simulink model "gate_V37_2016a_HIL_C"  (R2016a)
settings: Solver=FixedStepDiscrete  StartTime=0.0  StopTime=120  FixedStep=0.001  RelTol=1e-3
values from neighbouring files (symbols this model uses):
  CLOSE_SIDE_VALUE = single(4*ONE_SEC)    [GateParameter.m]
  CLOSED = uint8(12)    [GateParameter.m]
  CLOSING = uint8(13)    [GateParameter.m]
  CURRENT_STABLE_TIMER = uint8(200)    [GateParameter.m]
  CURRENT_THRESH = uint16(1000)    [GateParameter.m]
  INIT = uint8(15)    [GateParameter.m]
  MOVING_TIMER = uint32(60*ONE_SEC)    [GateParameter.m]
  OPEN = uint8(14)    [GateParameter.m]
  OPEN_SIDE_VALUE = uint16(4*ONE_SEC)    [GateParameter.m]
  OPENING = uint8(11)    [GateParameter.m]
  OPENING_ACTIVE = uint8(1)    [GateParameter.m]
  STABLE_TIMER_VALUE = uint16(1*ONE_SEC)    [GateParameter.m]
undefined symbols (not defined in the file or in its folder): sigbuilder_block
libraries: simulink x9, arduinolib x7
(part 2 of 2)

== system gate_V37_2016a_HIL_C/Gate/Subsystem/C_HardwareOutputWrapper_RF/MotorHandler ==
  leftMotor : Inport
  rightMotor : Inport  Port=2
  gateState : Inport  Port=3
  CLOSED : Constant  Value=CLOSED  SampleTime=-1
  CLOSING : Constant  Value=CLOSING  SampleTime=-1
  MotorsHandler : Stateflow chart  TreatAsAtomicUnit=on
  OPEN : Constant  Value=OPEN  SampleTime=-1
  OPENING : Constant  Value=OPENING  SampleTime=-1
  OPENING1 : Constant  Value=INIT  SampleTime=-1
  LeftPin : Outport
  ComPin : Outport  Port=2
  RightPin : Outport  Port=3
  wires:
  OPENING1/1 -> MotorsHandler/8(INIT)
  leftMotor/1 -> MotorsHandler/1(leftMotor)
  gateState/1 -> MotorsHandler/7(gateState)
  MotorsHandler/3(RightMotorPin_BOOL) -> RightPin/1
  MotorsHandler/2(ComMotorPin_BOOL) -> ComPin/1
  MotorsHandler/1(LeftMotorPin_BOOL) -> LeftPin/1
  OPENING/1 -> MotorsHandler/6(OPENING)
  OPEN/1 -> MotorsHandler/5(OPEN)
  CLOSING/1 -> MotorsHandler/4(CLOSING)
  CLOSED/1 -> MotorsHandler/3(CLOSED)
  rightMotor/1 -> MotorsHandler/2(rightMotor)

== system gate_V37_2016a_HIL_C/Gate/Subsystem/CurrentFilter/Subsystem ==
  rightCurrentRaw : Inport
  leftCurrentRaw : Inport  Port=2
  Data Type Conversion : DataTypeConversion  OutDataTypeStr=uint16
  Data Type Conversion1 : DataTypeConversion  OutDataTypeStr=uint16
  Logical Operator : Logic  Operator=OR  AllPortsSameDT=off  OutDataTypeStr=boolean
  S-Function Builder2 : S-Function  Commented=on  InitFcn=try, set_param(gcb,'FunctionName','printingBlockMotor'), end  FunctionName=printingBlockMotor  SFunctionModules=printingBlockMotor_wrapper  SFunctionDeploymentMode=off  EnableBusSupport=off  mask(S-Function Builder){}
      mask help: Simulink:masks:SFunctionBuilder_MD
      mask init: same as gate_V37_2016a_HIL_C/Gate/HardwareInputWrapper/HIL_B/S-Function Builder1
      settings: CopyFcn=set_param(gcbh,'LinkStatus','none')  PreSaveFcn=try, set_param(gcb,'FunctionName','printingBlockMotor'), end  OpenFcn=sfunctionwizard(gcbh)
      mask Display: plot(val(:,1),val(:,2)),disp(sys), port_label('input',1,'u0'), port_label('input',2,'u1')
  Unit Delay : UnitDelay  SampleTime=-1
  Unit Delay1 : UnitDelay  SampleTime=-1
  WRONG_PASSWORD : Constant  Value=CURRENT_STABLE_TIMER  SampleTime=-1
  WRONG_PASSWORD1 : Constant  Value=CURRENT_STABLE_TIMER  SampleTime=-1
  WRONG_PASSWORD2 : Constant  Value=CURRENT_THRESH  SampleTime=-1
  WRONG_PASSWORD3 : Constant  Value=CURRENT_THRESH  SampleTime=-1
  leftSquareWaveDetector : Stateflow chart  TreatAsAtomicUnit=on
  rightSquareWaveDetector : Stateflow chart  TreatAsAtomicUnit=on
  current : Outport
  wires:
  WRONG_PASSWORD3/1 -> leftSquareWaveDetector/4(CURRENT_THRESH)
  WRONG_PASSWORD2/1 -> rightSquareWaveDetector/4(CURRENT_THRESH)
  WRONG_PASSWORD1/1 -> leftSquareWaveDetector/3(CURRENT_STABLE_TIMER)
  WRONG_PASSWORD/1 -> rightSquareWaveDetector/3(CURRENT_STABLE_TIMER)
  Data Type Conversion1/1 -> S-Function Builder2/2
  Data Type Conversion/1 -> S-Function Builder2/1
  Logical Operator/1 -> current/1
  leftSquareWaveDetector/1(currentValue) -> Data Type Conversion1/1, Logical Operator/2
  rightSquareWaveDetector/1(currentValue) -> Logical Operator/1, Data Type Conversion/1
  Unit Delay1/1 -> leftSquareWaveDetector/2(CurrentAnalogInOld)
  leftCurrentRaw/1 -> leftSquareWaveDetector/1(CurrentAnalogIn), Unit Delay1/1
  Unit Delay/1 -> rightSquareWaveDetector/2(CurrentAnalogInOld)
  rightCurrentRaw/1 -> Unit Delay/1, rightSquareWaveDetector/1(CurrentAnalogIn)

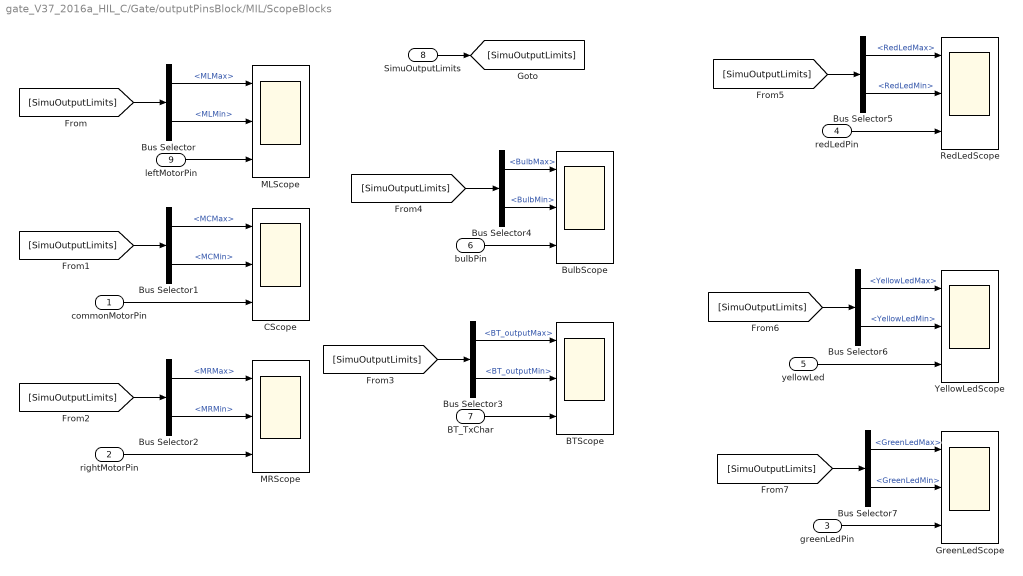
== system gate_V37_2016a_HIL_C/Gate/outputPinsBlock/MIL/ScopeBlocks ==
  commonMotorPin : Inport
  rightMotorPin : Inport  Port=2
  greenLedPin : Inport  Port=3
  redLedPin : Inport  Port=4
  yellowLed : Inport  Port=5
  bulbPin : Inport  Port=6
  BT_TxChar : Inport  Port=7
  SimuOutputLimits : Inport  Port=8
  leftMotorPin : Inport  Port=9
  BTScope : Scope  NumInputPorts=3
  BulbScope : Scope  NumInputPorts=3
  Bus Selector : BusSelector  OutputSignals=MLMax,MLMin
  Bus Selector1 : BusSelector  OutputSignals=MCMax,MCMin
  Bus Selector2 : BusSelector  OutputSignals=MRMax,MRMin
  Bus Selector3 : BusSelector  OutputSignals=BT_outputMax,BT_outputMin
  Bus Selector4 : BusSelector  OutputSignals=BulbMax,BulbMin
  Bus Selector5 : BusSelector  OutputSignals=RedLedMax,RedLedMin
  Bus Selector6 : BusSelector  OutputSignals=YellowLedMax,YellowLedMin
  Bus Selector7 : BusSelector  OutputSignals=GreenLedMax,GreenLedMin
  CScope : Scope  NumInputPorts=3
  GreenLedScope : Scope  NumInputPorts=3
  MLScope : Scope  NumInputPorts=3
  MRScope : Scope  NumInputPorts=3
  RedLedScope : Scope  NumInputPorts=3
  YellowLedScope : Scope  NumInputPorts=3
  wires:
  rightMotorPin/1 -> MRScope/3
  commonMotorPin/1 -> CScope/3
  bulbPin/1 -> BulbScope/3
  greenLedPin/1 -> GreenLedScope/3
  yellowLed/1 -> YellowLedScope/3
  redLedPin/1 -> RedLedScope/3
  BT_TxChar/1 -> BTScope/3
  SimuOutputLimits/1 -> [SimuOutputLimits]
  [SimuOutputLimits] -> Bus Selector/1
  leftMotorPin/1 -> MLScope/3
  Bus Selector/1 -> MLScope/1  "<MLMax>"
  Bus Selector/2 -> MLScope/2  "<MLMin>"
  [SimuOutputLimits] -> Bus Selector1/1
  Bus Selector1/1 -> CScope/1  "<MCMax>"
  Bus Selector1/2 -> CScope/2  "<MCMin>"
  [SimuOutputLimits] -> Bus Selector2/1
  Bus Selector2/1 -> MRScope/1  "<MRMax>"
  Bus Selector2/2 -> MRScope/2  "<MRMin>"
  [SimuOutputLimits] -> Bus Selector3/1
  Bus Selector3/1 -> BTScope/1  "<BT_outputMax>"
  Bus Selector3/2 -> BTScope/2  "<BT_outputMin>"
  [SimuOutputLimits] -> Bus Selector4/1
  Bus Selector4/1 -> BulbScope/1  "<BulbMax>"
  Bus Selector4/2 -> BulbScope/2  "<BulbMin>"
  [SimuOutputLimits] -> Bus Selector5/1
  Bus Selector5/1 -> RedLedScope/1  "<RedLedMax>"
  Bus Selector5/2 -> RedLedScope/2  "<RedLedMin>"
  [SimuOutputLimits] -> Bus Selector6/1
  Bus Selector6/1 -> YellowLedScope/1  "<YellowLedMax>"
  Bus Selector6/2 -> YellowLedScope/2  "<YellowLedMin>"
  [SimuOutputLimits] -> Bus Selector7/1
  Bus Selector7/1 -> GreenLedScope/1  "<GreenLedMax>"
  Bus Selector7/2 -> GreenLedScope/2  "<GreenLedMin>"

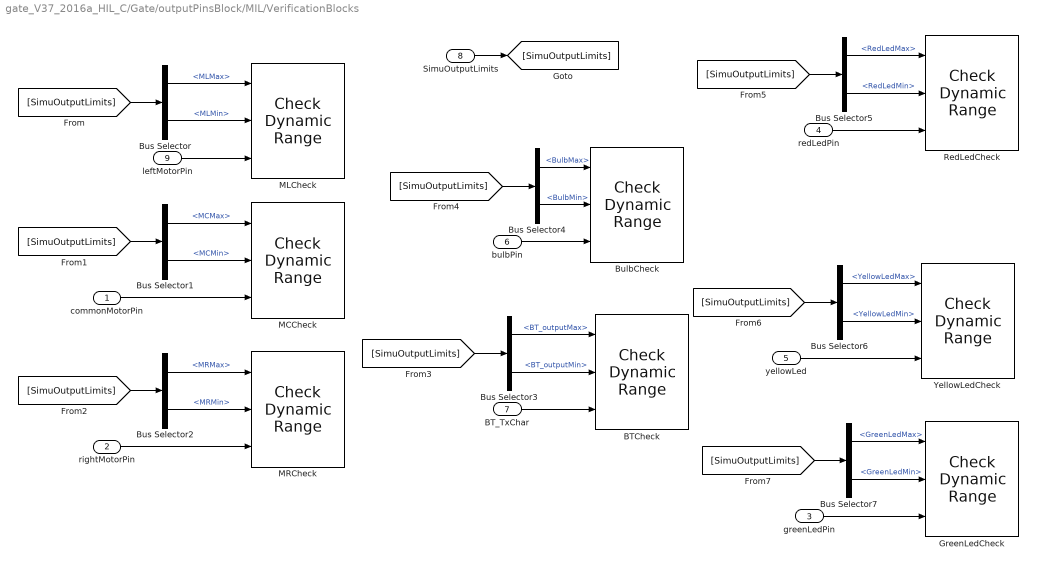
== system gate_V37_2016a_HIL_C/Gate/outputPinsBlock/MIL/VerificationBlocks  ==
  commonMotorPin : Inport
  rightMotorPin : Inport  Port=2
  greenLedPin : Inport  Port=3
  redLedPin : Inport  Port=4
  yellowLed : Inport  Port=5
  bulbPin : Inport  Port=6
  BT_TxChar : Inport  Port=7
  SimuOutputLimits : Inport  Port=8
  leftMotorPin : Inport  Port=9
  BTCheck : simulink/Model Verification/Check  Dynamic Range  Description=min < sig < max  enabled=on  stopWhenAssertionFail=on  export=off  icon=graphic
  BulbCheck : simulink/Model Verification/Check  Dynamic Range  Description=min < sig < max  enabled=on  stopWhenAssertionFail=on  export=off  icon=graphic
  Bus Selector : BusSelector  OutputSignals=MLMax,MLMin
  Bus Selector1 : BusSelector  OutputSignals=MCMax,MCMin
  Bus Selector2 : BusSelector  OutputSignals=MRMax,MRMin
  Bus Selector3 : BusSelector  OutputSignals=BT_outputMax,BT_outputMin
  Bus Selector4 : BusSelector  OutputSignals=BulbMax,BulbMin
  Bus Selector5 : BusSelector  OutputSignals=RedLedMax,RedLedMin
  Bus Selector6 : BusSelector  OutputSignals=YellowLedMax,YellowLedMin
  Bus Selector7 : BusSelector  OutputSignals=GreenLedMax,GreenLedMin
  GreenLedCheck : simulink/Model Verification/Check  Dynamic Range  Description=min < sig < max  enabled=on  stopWhenAssertionFail=on  export=off  icon=graphic
  MCCheck : simulink/Model Verification/Check  Dynamic Range  Description=min < sig < max  enabled=on  stopWhenAssertionFail=on  export=off  icon=graphic
  MLCheck : simulink/Model Verification/Check  Dynamic Range  Description=min < sig < max  enabled=on  stopWhenAssertionFail=on  export=off  icon=graphic
  MRCheck : simulink/Model Verification/Check  Dynamic Range  Description=min < sig < max  enabled=on  stopWhenAssertionFail=on  export=off  icon=graphic
  RedLedCheck : simulink/Model Verification/Check  Dynamic Range  Description=min < sig < max  enabled=on  stopWhenAssertionFail=on  export=off  icon=graphic
  YellowLedCheck : simulink/Model Verification/Check  Dynamic Range  Description=min < sig < max  enabled=on  stopWhenAssertionFail=on  export=off  icon=graphic
  wires:
  rightMotorPin/1 -> MRCheck/3
  commonMotorPin/1 -> MCCheck/3
  bulbPin/1 -> BulbCheck/3
  greenLedPin/1 -> GreenLedCheck/3
  yellowLed/1 -> YellowLedCheck/3
  redLedPin/1 -> RedLedCheck/3
  BT_TxChar/1 -> BTCheck/3
  SimuOutputLimits/1 -> [SimuOutputLimits]
  [SimuOutputLimits] -> Bus Selector/1
  leftMotorPin/1 -> MLCheck/3
  Bus Selector/1 -> MLCheck/1  "<MLMax>"
  Bus Selector/2 -> MLCheck/2  "<MLMin>"
  [SimuOutputLimits] -> Bus Selector1/1
  Bus Selector1/1 -> MCCheck/1  "<MCMax>"
  Bus Selector1/2 -> MCCheck/2  "<MCMin>"
  [SimuOutputLimits] -> Bus Selector2/1
  Bus Selector2/1 -> MRCheck/1  "<MRMax>"
  Bus Selector2/2 -> MRCheck/2  "<MRMin>"
  [SimuOutputLimits] -> Bus Selector3/1
  Bus Selector3/1 -> BTCheck/1  "<BT_outputMax>"
  Bus Selector3/2 -> BTCheck/2  "<BT_outputMin>"
  [SimuOutputLimits] -> Bus Selector4/1
  Bus Selector4/1 -> BulbCheck/1  "<BulbMax>"
  Bus Selector4/2 -> BulbCheck/2  "<BulbMin>"
  [SimuOutputLimits] -> Bus Selector5/1
  Bus Selector5/1 -> RedLedCheck/1  "<RedLedMax>"
  Bus Selector5/2 -> RedLedCheck/2  "<RedLedMin>"
  [SimuOutputLimits] -> Bus Selector6/1
  Bus Selector6/1 -> YellowLedCheck/1  "<YellowLedMax>"
  Bus Selector6/2 -> YellowLedCheck/2  "<YellowLedMin>"
  [SimuOutputLimits] -> Bus Selector7/1
  Bus Selector7/1 -> GreenLedCheck/1  "<GreenLedMax>"
  Bus Selector7/2 -> GreenLedCheck/2  "<GreenLedMin>"

== system gate_V37_2016a_HIL_C/Gate/HardwareInputWrapper/HIL_B/EEPROM/EEPROM_UPDATE ==
  clearReq : Inport
  readReq : Inport  Port=2
  writeReq : Inport  Port=3
  passwordIn : Inport  Port=4
  passAssignedIn : Inport  Port=5
  S-Function Builder : S-Function  InitFcn=try, set_param(gcb,'FunctionName','myEEPROM_Handler'), end  FunctionName=myEEPROM_Handler  SFunctionModules=myEEPROM_Handler_wrapper  SFunctionDeploymentMode=off  EnableBusSupport=off  mask(S-Function Builder){}
      mask help: Simulink:masks:SFunctionBuilder_MD
      mask init: same as gate_V37_2016a_HIL_C/Gate/HardwareInputWrapper/HIL_B/S-Function Builder1
      settings: CopyFcn=set_param(gcbh,'LinkStatus','none')  PreSaveFcn=try, set_param(gcb,'FunctionName','myEEPROM_Handler'), end  OpenFcn=sfunctionwizard(gcbh)
      mask Display: plot(val(:,1),val(:,2)),disp(sys), port_label('input',1,'passwordIn'), port_label('input',2,'readReq'), port_label('input',3,'oldReadReq'), port_label('input',4,'clearReq'), port_label('input',5,'oldClearReq'), port_label('input',6,'writeReq'), port_label('input',7,'oldWriteReq'), port_label('input',8,'passAssignedIn'), port_label('output',1,'passwordOut'), port_label('output',2,'readComplete'), port_label('output',3,'writeComplete'), port_label('output',4,'clearComplete'), port_label('output',5,'passAssignedOut')
  Unit Delay : UnitDelay  SampleTime=-1
  Unit Delay1 : UnitDelay  SampleTime=-1
  Unit Delay2 : UnitDelay  SampleTime=-1
  readComplete : Outport
  writeComplete : Outport  Port=2
  clearComplete : Outport  Port=3
  passwordOut : Outport  Port=4
  passAssignedOut : Outport  Port=5
  wires:
  S-Function Builder/5 -> passAssignedOut/1
  S-Function Builder/1 -> passwordOut/1
  Unit Delay1/1 -> S-Function Builder/5
  Unit Delay2/1 -> S-Function Builder/7
  writeReq/1 -> S-Function Builder/6, Unit Delay2/1
  passAssignedIn/1 -> S-Function Builder/8
  clearReq/1 -> S-Function Builder/4, Unit Delay1/1
  Unit Delay/1 -> S-Function Builder/3
  readReq/1 -> S-Function Builder/2, Unit Delay/1
  passwordIn/1 -> S-Function Builder/1
  S-Function Builder/4 -> clearComplete/1
  S-Function Builder/2 -> readComplete/1
  S-Function Builder/3 -> writeComplete/1

== stateflow chart gate_V37_2016a_HIL_C/Gate/Subsystem/CurrentFilter/Subsystem/leftSquareWaveDetector ==
  state Running
    entry:
    counterHigh = 0;
    counterLow= 0;
    currentValue = 1;
    state High
      entry:
      counterLow = 0;
    state Low
      entry:
      counterHigh = 0;
    (default) -> High
    High -> High [order 2] : [CurrentAnalogIn > CURRENT_THRESH]
        | /counterHigh++;
    High -> Low [order 1] : [CurrentAnalogIn < CURRENT_THRESH]
    Low -> High [order 1] : [CurrentAnalogIn > CURRENT_THRESH]
    Low -> Low [order 2] : [CurrentAnalogIn < CURRENT_THRESH]
        | /counterLow++;
  state Stop
    entry:
    currentValue = 0;
    stableCounter = 0;
  (default) -> Stop
  Stop -> Running : [((CurrentAnalogInOld > CURRENT_THRESH)...
      |  && (CurrentAnalogIn < CURRENT_THRESH)) ...
      | || ((CurrentAnalogInOld < CURRENT_THRESH)...
      |  && (CurrentAnalogIn > CURRENT_THRESH))]
  Running -> Stop : [(counterHigh >= CURRENT_STABLE_TIMER)...
      |  || (counterLow >= CURRENT_STABLE_TIMER)]
  data: CurrentAnalogIn input; currentValue output uint8; counterHigh local uint16; counterLow local uint16; stableCounter local uint8; CurrentAnalogInOld input; CURRENT_STABLE_TIMER input; CURRENT_THRESH input

== stateflow chart gate_V37_2016a_HIL_C/Gate/HardwareInputWrapper/HIL_B/EEPROM/EEPROM_Control ==
  state Idle
  state sendData
    entry:
    readReq = true;
  state Read
    entry:
    readReq = false;
  state write
    entry:
    writeReq = true;
  state clear
    entry:
    clearReq = true;
  Read -> sendData
  sendData -> Idle : [readComplete]/readReq = false;
  clear -> Idle [order 2] : [(hasChanged(passSw))...
      |  && (passSw == false)...
      | && (passAssigned == false)]
      | /clearReq = false;
  Idle -> clear : [(hasChanged(passSw))...
      |  && (passSw == true)]
  (default) -> Read
  write -> Read : [writeComplete]/writeReq = false;
  clear -> write [order 1] : [(hasChanged(passSw))...
      |  && (passSw == false)...
      | && (passAssigned == true)]
      | /clearReq = false;
  data: passSw input; passAssigned input; readComplete input; writeComplete input; clearReq output boolean =false; readReq output boolean =false; writeReq output boolean =false

== stateflow chart gate_V37_2016a_HIL_C/Gate/HardwareInputWrapper/HIL_B/currentSensorWrapperOld/SquareWaveDetector ==
  state Running
    entry:
    counterHigh = 0;
    counterLow= 0;
    currentValue = 1;
    state High
    state Low
    (default) -> High
    High -> High [order 2] : [CurrentAnalogIn > 1000]
        | /counterHigh++;
    High -> Low [order 1] : [CurrentAnalogIn < 1000]
    Low -> High [order 1] : [CurrentAnalogIn > 1000]
    Low -> Low [order 2] : [CurrentAnalogIn < 1000]
        | /counterLow++
  state Stop
    entry:
    currentValue = 0;
  (default) -> Stop
  Stop -> Running : [CurrentAnalogIn > 1000]
  Running -> Stop : [(counterHigh >= 200)...
      |  || (counterLow >= 200)]
  data: CurrentAnalogIn input; currentValue output uint8; counterHigh local uint16; counterLow local uint16

== stateflow chart gate_V37_2016a_HIL_C/Gate/Subsystem/C_HardwareOutputWrapper_RF/MotorHandler/MotorsHandler ==
  (default) -> (junction 146) : /* updating motor Pins Switch case */
  (junction 146) -> (junction 145) [order 1] : [(leftMotor == OPENING) && ((rightMotor == CLOSED) || (rightMotor == OPEN))]
  (junction 145) -> (junction 161) : {LeftMotorPin_BOOL = true;...
      | ComMotorPin_BOOL = true;...
      | RightMotorPin_BOOL = false;}
  (junction 146) -> (junction 148) [order 2]
  (junction 148) -> (junction 147) [order 1] : [(leftMotor == CLOSING) && ((rightMotor == CLOSED) || (rightMotor == OPEN))]
  (junction 147) -> (junction 162) : {LeftMotorPin_BOOL = false;...
      | ComMotorPin_BOOL = false;...
      |  RightMotorPin_BOOL = true;}
  (junction 148) -> (junction 151) [order 2]
  (junction 151) -> (junction 150) [order 1] : [(rightMotor == CLOSING) && ((leftMotor == CLOSED) || (leftMotor == OPEN))]
  (junction 150) -> (junction 163) : {LeftMotorPin_BOOL = true;...
      | ComMotorPin_BOOL = false;...
      | RightMotorPin_BOOL = false;}
  (junction 151) -> (junction 152) [order 2]
  (junction 152) -> (junction 149) [order 1] : [(rightMotor == OPENING) && ((leftMotor == CLOSED) || (leftMotor == OPEN))]
  (junction 149) -> (junction 169) : {LeftMotorPin_BOOL = false;...
      | ComMotorPin_BOOL = true;...
      | RightMotorPin_BOOL = true;}
  (junction 152) -> (junction 154) [order 2]
  (junction 154) -> (junction 153) [order 1] : [(rightMotor == OPENING) && (leftMotor == OPENING)]
  (junction 153) -> (junction 168) : {LeftMotorPin_BOOL = true;...
      | ComMotorPin_BOOL = true;...
      | RightMotorPin_BOOL = true;}
  (junction 154) -> (junction 155) [order 2]
  (junction 155) -> (junction 156) [order 1] : [(rightMotor == CLOSING) && (leftMotor == CLOSING)]
  (junction 156) -> (junction 167) : {LeftMotorPin_BOOL = false;...
      | ComMotorPin_BOOL = false;...
      | RightMotorPin_BOOL = false;}
  (junction 155) -> (junction 157) [order 2]
  (junction 157) -> (junction 158) [order 1] : [((rightMotor == CLOSED) || (rightMotor == OPEN)) ...
      | && ((leftMotor == CLOSED) || (leftMotor == OPEN))]
  (junction 157) -> (junction 159) [order 2]
  (junction 158) -> (junction 166) : {LeftMotorPin_BOOL = false;...
      | ComMotorPin_BOOL = true;...
      | RightMotorPin_BOOL = false;}
  (junction 159) -> (junction 160) [order 1] : [(gateState == CLOSED) || (gateState == OPEN) || (gateState == INIT)]
  (junction 159) -> (junction 164) [order 2]
  (junction 160) -> (junction 165) : {LeftMotorPin_BOOL = false;...
      | ComMotorPin_BOOL = true;...
      | RightMotorPin_BOOL = false;}
  (junction 161) -> (junction 162)
  (junction 162) -> (junction 163)
  (junction 163) -> (junction 169)
  (junction 165) -> (junction 164)
  (junction 166) -> (junction 165)
  (junction 167) -> (junction 166)
  (junction 168) -> (junction 167)
  (junction 169) -> (junction 168)
  (junction 164) -> (junction 170)
  data: leftMotor input; LeftMotorPin_BOOL output boolean =false; ComMotorPin_BOOL output boolean =true; RightMotorPin_BOOL output boolean =false; rightMotor input; CLOSED input; CLOSING input; OPEN input; OPENING input; gateState input; INIT input

== stateflow chart gate_V37_2016a_HIL_C/Gate/Subsystem/CurrentFilter/10msSmapler ==
  (default) -> (junction 2)
  (junction 2) -> (junction 3) : {counter_1ms++;}
  (junction 3) -> (junction 6) : [counter_1ms == 10]
      | {
      | triggerOn;
      | counter_1ms = 0;
      | }
  data: counter_1ms local double
  events: triggerOn output FUNCTION_CALL_EVENT

== stateflow chart gate_V37_2016a_HIL_C/Gate/Subsystem/CurrentFilter/Subsystem/rightSquareWaveDetector ==
  state Running
    entry:
    counterHigh = 0;
    counterLow= 0;
    currentValue = 1;
    state High
      entry:
      counterLow = 0;
    state Low
      entry:
      counterHigh = 0;
    (default) -> High
    High -> High [order 2] : [CurrentAnalogIn > CURRENT_THRESH]
        | /counterHigh++;
    High -> Low [order 1] : [CurrentAnalogIn < CURRENT_THRESH]
    Low -> High [order 1] : [CurrentAnalogIn > CURRENT_THRESH]
    Low -> Low [order 2] : [CurrentAnalogIn < CURRENT_THRESH]
        | /counterLow++;
  state Stop
    entry:
    currentValue = 0;
    stableCounter = 0;
  (default) -> Stop
  Stop -> Running : [((CurrentAnalogInOld > CURRENT_THRESH)...
      |  && (CurrentAnalogIn < CURRENT_THRESH)) ...
      | || ((CurrentAnalogInOld < CURRENT_THRESH)...
      |  && (CurrentAnalogIn > CURRENT_THRESH))]
  Running -> Stop : [(counterHigh >= CURRENT_STABLE_TIMER)...
      |  || (counterLow >= CURRENT_STABLE_TIMER)]
  data: CurrentAnalogIn input; currentValue output uint8; counterHigh local uint16; counterLow local uint16; stableCounter local uint8; CurrentAnalogInOld input; CURRENT_STABLE_TIMER input; CURRENT_THRESH input

== stateflow chart gate_V37_2016a_HIL_C/Gate/Subsystem/C_Variant_RF/GateLogicStatemachine ==
  state init
    entry:
    gotoReady = false;
    bulbPin_BOOL = 0;
    leftMotor = CLOSING;
    rightMotor = CLOSING;
    gateState = INIT;
  state opening
    entry:
    bulbPin_BOOL = true;
    gateState = OPENING;
    state openLeftSide
      entry:
      leftMotor = OPENING;
      rightMotor = CLOSED;
    state openRightSide
      entry:
      rightMotor = OPENING;
    (default) -> openLeftSide
    openLeftSide -> openRightSide : [after(OPEN_SIDE_VALUE, tick)]
  state closed
    entry:
    bulbPin_BOOL = false;
    leftMotor = CLOSING;
    rightMotor = CLOSING;
    openingActive = 0;
    gateState = CLOSED;
    closingTimer = 0;
    state openingGate
      entry:
      openingActive = OPENING_ACTIVE;
    state MonitorInput
    (default) -> MonitorInput
    MonitorInput -> openingGate [order 1] : [(RFPressedOut_UINT8 == true) && hasChanged(RFPressedOut_UINT8)]
    MonitorInput -> openingGate [order 2] : [((passSwitch == true) && hasChanged(passSwitch))]
  state closing
    entry:
    gateState = CLOSING;
    state closingRightSide
      entry:
      rightMotor = CLOSING;
    state closingLeftSide
      entry:
      leftMotor = CLOSING;
    (default) -> closingRightSide
    closingRightSide -> closingLeftSide : [after(CLOSE_SIDE_VALUE, tick)]
  state open
    entry:
    gateState = OPEN;
    state gateOpen
      entry:
      closing_active = false;
      leftMotor = OPEN;
      rightMotor = OPEN;
    state closing
      entry:
      closing_active = true;
    (default) -> gateOpen
    gateOpen -> open.closing [order 2] : [((passSwitch == true) && hasChanged(passSwitch))]
    gateOpen -> (junction 385) [order 1] : [(hasChanged(RFPressedOut_UINT8))]
    (junction 385) -> open.closing : [(RFPressedOut_UINT8 == true)]
  (default) -> init
  closed -> opening : [openingActive == OPENING_ACTIVE]
  init -> closed
  closing -> closed [order 2] : [(current_sensor == 0)...
      | &&(after(CLOSE_SIDE_VALUE + STABLE_TIMER_VALUE, tick))]
  closing -> closed [order 3] : [after(MOVING_TIMER, tick)]
  (junction 383) -> closed : [(after(CLOSE_SIDE_VALUE...
      |  + STABLE_TIMER_VALUE, tick))]
  opening -> (junction 381) [order 1] : [(((RFPressedOut_UINT8 == true)...
      | && hasChanged(RFPressedOut_UINT8)) ...
      | || ((passSwitch == true)...
      |  && hasChanged(passSwitch)))]
  opening -> open [order 3] : [after(MOVING_TIMER, tick)]
  opening -> open [order 2] : [(current_sensor == 0)...
      | && (after(OPEN_SIDE_VALUE + STABLE_TIMER_VALUE, tick))]
  (junction 381) -> open : [(after(OPEN_SIDE_VALUE...
      |  + STABLE_TIMER_VALUE, tick))]
  closing -> (junction 383) [order 1] : [(((RFPressedOut_UINT8 == true)...
      | &&hasChanged(RFPressedOut_UINT8)) ...
      | || ((passSwitch == true)...
      |  && hasChanged(passSwitch)))]
  open -> closing : [closing_active == true]
  data: RFPressedOut_UINT8 input; openingActive local uint8; passSwitch input; closing_active local uint8; current_sensor input; leftMotor output uint8 =0; rightMotor output uint8 =0; bulbPin_BOOL output boolean; OPENING_ACTIVE input =2; OPEN_SIDE_VALUE input =20; CLOSE_SIDE_VALUE input =20; OPENING input =2; CLOSED input =1; CLOSING input =4; OPEN input =3; gotoReady local boolean; gateState output uint8; closingTimer local uint32; INIT input; MOVING_TIMER input; STABLE_TIMER_VALUE input
